simulink model "os_polyphase_synth_4096c"  (R2021a)
settings: Solver=VariableStepAuto  StartTime=0.0  StopTime=2304  RelTol=1e-3
undefined symbols (not defined in the file or in its folder): A, B, C, pfb_coeff_gen_calc
libraries: xbsIndex_r4 x22061, casper_library_misc x266, casper_library_delays x127, casper_library_multipliers x88
(part 109 of 241)

== system os_polyphase_synth_4096c/pfb_fir/pol1_in1_tap88 ==
  din : Inport
  sync : Inport  Port=2
  coeff : Inport  Port=3
  taps : Inport  Port=4
  Convert : xbsIndex_r4/Convert  gui_display_data_type=Fixed-point  arith_type="Signed  (2's comp)"  n_bits=36+3  bin_pt=34  float_type=Single  exp_bits=8  fraction_bits=24  quantization=Truncate  overflow=Wrap  en=off  latency=0  pipeline=off  dbl_ovrd=off  block_type=convert
  Convert1 : xbsIndex_r4/Convert  gui_display_data_type=Fixed-point  arith_type="Signed  (2's comp)"  n_bits=36+3  bin_pt=34  float_type=Single  exp_bits=8  fraction_bits=24  quantization=Truncate  overflow=Wrap  en=off  latency=0  pipeline=off  dbl_ovrd=off  block_type=convert
  DSP Macro 1.0 : xbsIndex_r4/DSP Macro 1.0   dsptype=Target XtremeDSP Slice: DSP48E2  instruction_list=A*B+C  AvailableInstructions=|  show_filtered=off  pipeline_options=Expert  dspimage=?  tier_1=off  tier_2=off  tier_3=off  tier_4=off  tier_5=off  tier_6=off  dreg_1=off  dreg_2=off  dreg_3=off  areg_1=off  areg_2=off  areg_3=on  areg_4=on  breg_1=off  breg_2=off  breg_3=off  breg_4=on  mreg_5=on  concatreg_3=off  concatreg_4=off  concatreg_5=off  creg_1=off  creg_2=off  creg_3=off  creg_4=off  creg_5=on  preg_6=off  cinreg_1=off  cinreg_2=off  cinreg_3=off  cinreg_4=off  cinreg_5=off  opreg_1=off  opreg_2=off  opreg_3=off  opreg_4=off  opreg_5=off  output_properties=Full_Precision  p_width=37  p_binarywidth=34  has_acout=off  has_bcout=off  has_pcout=off  has_carryout=off  has_carrycascout=off  has_ce=off  has_d_ce=off  has_a_ce=off  has_b_ce=off  has_concat_ce=off  has_c_ce=off  has_m_ce=off  has_p_ce=off  has_sel_ce=off  has_sclr=off  has_d_sclr=off  has_a_sclr=off  has_b_sclr=off  has_concat_sclr=off  has_c_sclr=off  has_m_sclr=off  has_p_sclr=off  has_sel_sclr=off  use_dsp48=on  simulation_type=xsim  gui_behaviour=Sysgen  instruction1='#'  ip_name=DSP Macro  ip_version=1.0  dsptool_ready=true  ipcore_usecache=true  ipcore_useipmodelcache=true  wrapper_available=true  port_translation_map={ 'ce' => 'en', 'sclr' => 'rst'}  ipcore_latency_function='has_combinational'  structural_sim=false  ipcore_verbose=false  ipcore_port_config={ 'carryin' => { 'arith' => 'ufix', 'binpt' => 0.00000000000, 'width' => 1.00000000000}, 'carrycascin' => { 'arith' => 'ufix', 'binpt' => 0.00000000000, 'width' => 1.00000000000}, 'carrycascout' => { 'arith' => 'ufix', 'binpt' => 0.00000000000, 'width' => 1.00000000000}, 'carryout' => { 'arith' => 'ufix', 'binpt' => 0.00000000000, 'width' => 1.00000000000}}  ipcore_xco_need_fpga_part=true  block_type=dsp_macro_v1_0
      | #
      | (A+D)
      | (A+D)*(A+D)
      | (A+D)*(A+D)+C
      | (A+D)*(A+D)+C+C
      | (A+D)*(A+D)+C+C+CARRYIN
      | (A+D)*(A+D)+C+CARRYIN
      | (A+D)*(A+D)+C+P
      | (A+D)*(A+D)+C+P+CARRYIN
      | (A+D)*(A+D)+C+P>>17
      | (A+D)*(A+D)+C+P>>17+CARRYIN
      | (A+D)*(A+D)+C+PCIN
      | (A+D)*(A+D)+C+PCIN+CARRYIN
      | (A+D)*(A+D)+C+PCIN>>17
      | (A+D)*(A+D)+C+PCIN>>17+CARRYIN
      | (A+D)*(A+D)+C-C
      | (A+D)*(A+D)+C-P
      | (A+D)*(A+D)+C-P>>17
      | (A+D)*(A+D)+C-PCIN
      | (A+D)*(A+D)+C-PCIN>>17
      | (A+D)*(A+D)+CARRYIN
      | (A+D)*(A+D)+P
      | (A+D)*(A+D)+P+C
      | (A+D)*(A+D)+P+C+CARRYIN
      | (A+D)*(A+D)+P+CARRYIN
      | (A+D)*(A+D)+P+P
      | (A+D)*(A+D)+P+P+CARRYIN
      | (A+D)*(A+D)+P+P>>17
      | (A+D)*(A+D)+P+P>>17+CARRYIN
      | (A+D)*(A+D)+P+PCIN
      | (A+D)*(A+D)+P+PCIN+CARRYIN
      | (A+D)*(A+D)+P+PCIN>>17
      | (A+D)*(A+D)+P+PCIN>>17+CARRYIN
      | (A+D)*(A+D)+P-C
      | (A+D)*(A+D)+P-P
      | (A+D)*(A+D)+P-P>>17
      | (A+D)*(A+D)+P-PCIN
      | (A+D)*(A+D)+P-PCIN>>17
      | (A+D)*(A+D)+P>>17
      | (A+D)*(A+D)+P>>17+C
      | (A+D)*(A+D)+P>>17+C+CARRYIN
      | (A+D)*(A+D)+P>>17+CARRYIN
      | (A+D)*(A+D)+P>>17+P
      | (A+D)*(A+D)+P>>17+P+CARRYIN
      | (A+D)*(A+D)+PCIN
      | (A+D)*(A+D)+PCIN+C
      | (A+D)*(A+D)+PCIN+C+CARRYIN
      | (A+D)*(A+D)+PCIN+CARRYIN
      | (A+D)*(A+D)+PCIN+P
      | (A+D)*(A+D)+PCIN+P+CARRYIN
      | (A+D)*(A+D)+PCIN>>17
      | (A+D)*(A+D)+PCIN>>17+C
      | (A+D)*(A+D)+PCIN>>17+C+CARRYIN
      | (A+D)*(A+D)+PCIN>>17+CARRYIN
      | (A+D)*(A+D)+PCIN>>17+P
      | (A+D)*(A+D)+PCIN>>17+P+CARRYIN
      | (A+D)*(A+D)-C
      | (A+D)*(A+D)-P
      | (A+D)*(A+D)-P>>17
      | (A+D)*(A+D)-PCIN
      | (A+D)*(A+D)-PCIN>>17
      | (A+D)*B
      | (A+D)*B+C
      | (A+D)*B+C+C
      | (A+D)*B+C+C+CARRYIN
      | (A+D)*B+C+CARRYIN
      | (A+D)*B+C+P
      | (A+D)*B+C+P+CARRYIN
      | (A+D)*B+C+P>>17
      | (A+D)*B+C+P>>17+CARRYIN
      | (A+D)*B+C+PCIN
      | (A+D)*B+C+PCIN+CARRYIN
      | (A+D)*B+C+PCIN>>17
      | (A+D)*B+C+PCIN>>17+CARRYIN
      | (A+D)*B+C-C
      | (A+D)*B+C-P
      | (A+D)*B+C-P>>17
      | (A+D)*B+C-PCIN
      | (A+D)*B+C-PCIN>>17
      | (A+D)*B+CARRYIN
      | (A+D)*B+P
      | (A+D)*B+P+C
      | (A+D)*B+P+C+CARRYIN
      | (A+D)*B+P+CARRYIN
      | (A+D)*B+P+P
      | (A+D)*B+P+P+CARRYIN
      | (A+D)*B+P+P>>17
      | (A+D)*B+P+P>>17+CARRYIN
      | (A+D)*B+P+PCIN
      | (A+D)*B+P+PCIN+CARRYIN
      | (A+D)*B+P+PCIN>>17
      | (A+D)*B+P+PCIN>>17+CARRYIN
      | (A+D)*B+P-C
      | (A+D)*B+P-P
      | (A+D)*B+P-P>>17
      | (A+D)*B+P-PCIN
      | (A+D)*B+P-PCIN>>17
      | (A+D)*B+P>>17
      | (A+D)*B+P>>17+C
      | (A+D)*B+P>>17+C+CARRYIN
      | (A+D)*B+P>>17+CARRYIN
      | (A+D)*B+P>>17+P
      | (A+D)*B+P>>17+P+CARRYIN
      | (A+D)*B+PCIN
      | (A+D)*B+PCIN+C
      | (A+D)*B+PCIN+C+CARRYIN
      | (A+D)*B+PCIN+CARRYIN
      | (A+D)*B+PCIN+P
      | (A+D)*B+PCIN+P+CARRYIN
      | (A+D)*B+PCIN>>17
      | (A+D)*B+PCIN>>17+C
      | (A+D)*B+PCIN>>17+C+CARRYIN
      | (A+D)*B+PCIN>>17+CARRYIN
      | (A+D)*B+PCIN>>17+P
      | (A+D)*B+PCIN>>17+P+CARRYIN
      | (A+D)*B-C
      | (A+D)*B-P
      | (A+D)*B-P>>17
      | (A+D)*B-PCIN
      | (A+D)*B-PCIN>>17
      | (A+D)*BCIN
      | (A+D)*BCIN+C
      | (A+D)*BCIN+C+C
      | (A+D)*BCIN+C+C+CARRYIN
      | (A+D)*BCIN+C+CARRYIN
      | (A+D)*BCIN+C+P
      | (A+D)*BCIN+C+P+CARRYIN
      | (A+D)*BCIN+C+P>>17
      | (A+D)*BCIN+C+P>>17+CARRYIN
      | (A+D)*BCIN+C+PCIN
      | (A+D)*BCIN+C+PCIN+CARRYIN
      | (A+D)*BCIN+C+PCIN>>17
      | (A+D)*BCIN+C+PCIN>>17+CARRYIN
      | (A+D)*BCIN+C-C
      | (A+D)*BCIN+C-P
      | (A+D)*BCIN+C-P>>17
      | (A+D)*BCIN+C-PCIN
      | (A+D)*BCIN+C-PCIN>>17
      | (A+D)*BCIN+CARRYIN
      | (A+D)*BCIN+P
      | (A+D)*BCIN+P+C
      | (A+D)*BCIN+P+C+CARRYIN
      | (A+D)*BCIN+P+CARRYIN
      | (A+D)*BCIN+P+P
      | (A+D)*BCIN+P+P+CARRYIN
      | (A+D)*BCIN+P+P>>17
      | (A+D)*BCIN+P+P>>17+CARRYIN
      | (A+D)*BCIN+P+PCIN
      | (A+D)*BCIN+P+PCIN+CARRYIN
      | (A+D)*BCIN+P+PCIN>>17
      | (A+D)*BCIN+P+PCIN>>17+CARRYIN
      | (A+D)*BCIN+P-C
      | (A+D)*BCIN+P-P
      | (A+D)*BCIN+P-P>>17
      | (A+D)*BCIN+P-PCIN
      | (A+D)*BCIN+P-PCIN>>17
      | (A+D)*BCIN+P>>17
      | (A+D)*BCIN+P>>17+C
      | (A+D)*BCIN+P>>17+C+CARRYIN
      | (A+D)*BCIN+P>>17+CARRYIN
      | (A+D)*BCIN+P>>17+P
      | (A+D)*BCIN+P>>17+P+CARRYIN
      | (A+D)*BCIN+PCIN
      | (A+D)*BCIN+PCIN+C
      | (A+D)*BCIN+PCIN+C+CARRYIN
      | (A+D)*BCIN+PCIN+CARRYIN
      | (A+D)*BCIN+PCIN+P
      | (A+D)*BCIN+PCIN+P+CARRYIN
      | (A+D)*BCIN+PCIN>>17
      | (A+D)*BCIN+PCIN>>17+C
      | (A+D)*BCIN+PCIN>>17+C+CARRYIN
      | (A+D)*BCIN+PCIN>>17+CARRYIN
      | (A+D)*BCIN+PCIN>>17+P
      | (A+D)*BCIN+PCIN>>17+P+CARRYIN
      | (A+D)*BCIN-C
      | (A+D)*BCIN-P
      | (A+D)*BCIN-P>>17
      | (A+D)*BCIN-PCIN
      | (A+D)*BCIN-PCIN>>17
      | (A+D)+C
      | (A+D)+C+C
      | (A+D)+C+C+CARRYIN
      | (A+D)+C+CARRYIN
      | (A+D)+C+P
      | (A+D)+C+P+CARRYIN
      | (A+D)+C+P>>17
      | (A+D)+C+P>>17+CARRYIN
      | (A+D)+C+PCIN
      | (A+D)+C+PCIN+CARRYIN
      | (A+D)+C+PCIN>>17
      | (A+D)+C+PCIN>>17+CARRYIN
      | (A+D)+C-C
      | (A+D)+C-P
      | (A+D)+C-P>>17
      | (A+D)+C-PCIN
      | (A+D)+C-PCIN>>17
      | (A+D)+CARRYIN
      | (A+D)+P
      | (A+D)+P+C
      | (A+D)+P+C+CARRYIN
      | (A+D)+P+CARRYIN
      | (A+D)+P+P
      | (A+D)+P+P+CARRYIN
      | (A+D)+P+P>>17
      | (A+D)+P+P>>17+CARRYIN
      | (A+D)+P+PCIN
      | (A+D)+P+PCIN+CARRYIN
      | (A+D)+P+PCIN>>17
      | (A+D)+P+PCIN>>17+CARRYIN
      | (A+D)+P-C
      | (A+D)+P-P
      | (A+D)+P-P>>17
      | (A+D)+P-PCIN
      | (A+D)+P-PCIN>>17
      | (A+D)+P>>17
      | (A+D)+P>>17+C
      | (A+D)+P>>17+C+CARRYIN
      | (A+D)+P>>17+CARRYIN
      | (A+D)+P>>17+P
      | (A+D)+P>>17+P+CARRYIN
      | (A+D)+PCIN
      | (A+D)+PCIN+C
      | (A+D)+PCIN+C+CARRYIN
      | (A+D)+PCIN+CARRYIN
      | (A+D)+PCIN+P
      | (A+D)+PCIN+P+CARRYIN
      | (A+D)+PCIN>>17
      | (A+D)+PCIN>>17+C
      | (A+D)+PCIN>>17+C+CARRYIN
      | (A+D)+PCIN>>17+CARRYIN
      | (A+D)+PCIN>>17+P
      | (A+D)+PCIN>>17+P+CARRYIN
      | (A+D)-C
      | (A+D)-P
      | (A+D)-P>>17
      | (A+D)-PCIN
      | (A+D)-PCIN>>17
      | (ACIN+D)
      | (ACIN+D)*(ACIN+D)
      | (ACIN+D)*(ACIN+D)+C
      | (ACIN+D)*(ACIN+D)+C+C
      | (ACIN+D)*(ACIN+D)+C+C+CARRYIN
      | (ACIN+D)*(ACIN+D)+C+CARRYIN
      | (ACIN+D)*(ACIN+D)+C+P
      | (ACIN+D)*(ACIN+D)+C+P+CARRYIN
      | (ACIN+D)*(ACIN+D)+C+P>>17
      | (ACIN+D)*(ACIN+D)+C+P>>17+CARRYIN
      | (ACIN+D)*(ACIN+D)+C+PCIN
      | (ACIN+D)*(ACIN+D)+C+PCIN+CARRYIN
      | (ACIN+D)*(ACIN+D)+C+PCIN>>17
      | (ACIN+D)*(ACIN+D)+C+PCIN>>17+CARRYIN
      | (ACIN+D)*(ACIN+D)+C-C
      | (ACIN+D)*(ACIN+D)+C-P
      | (ACIN+D)*(ACIN+D)+C-P>>17
      | (ACIN+D)*(ACIN+D)+C-PCIN
      | (ACIN+D)*(ACIN+D)+C-PCIN>>17
      | (ACIN+D)*(ACIN+D)+CARRYIN
      | (ACIN+D)*(ACIN+D)+P
      | (ACIN+D)*(ACIN+D)+P+C
      | (ACIN+D)*(ACIN+D)+P+C+CARRYIN
      | (ACIN+D)*(ACIN+D)+P+CARRYIN
      | (ACIN+D)*(ACIN+D)+P+P
      | (ACIN+D)*(ACIN+D)+P+P+CARRYIN
      | (ACIN+D)*(ACIN+D)+P+P>>17
      | (ACIN+D)*(ACIN+D)+P+P>>17+CARRYIN
      | (ACIN+D)*(ACIN+D)+P+PCIN
      | (ACIN+D)*(ACIN+D)+P+PCIN+CARRYIN
      | (ACIN+D)*(ACIN+D)+P+PCIN>>17
      | (ACIN+D)*(ACIN+D)+P+PCIN>>17+CARRYIN
      | (ACIN+D)*(ACIN+D)+P-C
      | (ACIN+D)*(ACIN+D)+P-P
      | (ACIN+D)*(ACIN+D)+P-P>>17
      | (ACIN+D)*(ACIN+D)+P-PCIN
      | (ACIN+D)*(ACIN+D)+P-PCIN>>17
      | (ACIN+D)*(ACIN+D)+P>>17
      | (ACIN+D)*(ACIN+D)+P>>17+C
      | (ACIN+D)*(ACIN+D)+P>>17+C+CARRYIN
      | (ACIN+D)*(ACIN+D)+P>>17+CARRYIN
      | (ACIN+D)*(ACIN+D)+P>>17+P
      | (ACIN+D)*(ACIN+D)+P>>17+P+CARRYIN
      | (ACIN+D)*(ACIN+D)+PCIN
      | (ACIN+D)*(ACIN+D)+PCIN+C
      | (ACIN+D)*(ACIN+D)+PCIN+C+CARRYIN
      | (ACIN+D)*(ACIN+D)+PCIN+CARRYIN
      | (ACIN+D)*(ACIN+D)+PCIN+P
      | (ACIN+D)*(ACIN+D)+PCIN+P+CARRYIN
      | (ACIN+D)*(ACIN+D)+PCIN>>17
      | (ACIN+D)*(ACIN+D)+PCIN>>17+C
      | (ACIN+D)*(ACIN+D)+PCIN>>17+C+CARRYIN
      | (ACIN+D)*(ACIN+D)+PCIN>>17+CARRYIN
      | (ACIN+D)*(ACIN+D)+PCIN>>17+P
      | (ACIN+D)*(ACIN+D)+PCIN>>17+P+CARRYIN
      | (ACIN+D)*(ACIN+D)-C
      | (ACIN+D)*(ACIN+D)-P
      | (ACIN+D)*(ACIN+D)-P>>17
      | (ACIN+D)*(ACIN+D)-PCIN
      | (ACIN+D)*(ACIN+D)-PCIN>>17
      | (ACIN+D)*B
      | (ACIN+D)*B+C
      | (ACIN+D)*B+C+C
      | (ACIN+D)*B+C+C+CARRYIN
      | (ACIN+D)*B+C+CARRYIN
      | (ACIN+D)*B+C+P
      | (ACIN+D)*B+C+P+CARRYIN
      | (ACIN+D)*B+C+P>>17
      | (ACIN+D)*B+C+P>>17+CARRYIN
      | (ACIN+D)*B+C+PCIN
      | (ACIN+D)*B+C+PCIN+CARRYIN
      | (ACIN+D)*B+C+PCIN>>17
      | (ACIN+D)*B+C+PCIN>>17+CARRYIN
      | (ACIN+D)*B+C-C
      | (ACIN+D)*B+C-P
      | (ACIN+D)*B+C-P>>17
      | (ACIN+D)*B+C-PCIN
      | (ACIN+D)*B+C-PCIN>>17
      | (ACIN+D)*B+CARRYIN
      | (ACIN+D)*B+P
      | (ACIN+D)*B+P+C
      | (ACIN+D)*B+P+C+CARRYIN
      | (ACIN+D)*B+P+CARRYIN
      | (ACIN+D)*B+P+P
      | (ACIN+D)*B+P+P+CARRYIN
      | (ACIN+D)*B+P+P>>17
      | (ACIN+D)*B+P+P>>17+CARRYIN
      | (ACIN+D)*B+P+PCIN
      | (ACIN+D)*B+P+PCIN+CARRYIN
      | (ACIN+D)*B+P+PCIN>>17
      | (ACIN+D)*B+P+PCIN>>17+CARRYIN
      | (ACIN+D)*B+P-C
      | (ACIN+D)*B+P-P
      | (ACIN+D)*B+P-P>>17
      | (ACIN+D)*B+P-PCIN
      | (ACIN+D)*B+P-PCIN>>17
      | (ACIN+D)*B+P>>17
      | (ACIN+D)*B+P>>17+C
      | (ACIN+D)*B+P>>17+C+CARRYIN
      | (ACIN+D)*B+P>>17+CARRYIN
      | (ACIN+D)*B+P>>17+P
      | (ACIN+D)*B+P>>17+P+CARRYIN
      | (ACIN+D)*B+PCIN
      | (ACIN+D)*B+PCIN+C
      | (ACIN+D)*B+PCIN+C+CARRYIN
      | (ACIN+D)*B+PCIN+CARRYIN
      | (ACIN+D)*B+PCIN+P
      | (ACIN+D)*B+PCIN+P+CARRYIN
      | (ACIN+D)*B+PCIN>>17
      | (ACIN+D)*B+PCIN>>17+C
      | (ACIN+D)*B+PCIN>>17+C+CARRYIN
      | (ACIN+D)*B+PCIN>>17+CARRYIN
      | (ACIN+D)*B+PCIN>>17+P
      | (ACIN+D)*B+PCIN>>17+P+CARRYIN
      | (ACIN+D)*B-C
      | (ACIN+D)*B-P
      | (ACIN+D)*B-P>>17
      | (ACIN+D)*B-PCIN
      | (ACIN+D)*B-PCIN>>17
      | (ACIN+D)*BCIN
      | (ACIN+D)*BCIN+C
      | (ACIN+D)*BCIN+C+C
      | (ACIN+D)*BCIN+C+C+CARRYIN
      | (ACIN+D)*BCIN+C+CARRYIN
      | (ACIN+D)*BCIN+C+P
      | (ACIN+D)*BCIN+C+P+CARRYIN
      | (ACIN+D)*BCIN+C+P>>17
      | (ACIN+D)*BCIN+C+P>>17+CARRYIN
      | (ACIN+D)*BCIN+C+PCIN
      | (ACIN+D)*BCIN+C+PCIN+CARRYIN
      | (ACIN+D)*BCIN+C+PCIN>>17
      | (ACIN+D)*BCIN+C+PCIN>>17+CARRYIN
      | (ACIN+D)*BCIN+C-C
      | (ACIN+D)*BCIN+C-P
      | (ACIN+D)*BCIN+C-P>>17
      | (ACIN+D)*BCIN+C-PCIN
      | (ACIN+D)*BCIN+C-PCIN>>17
      | (ACIN+D)*BCIN+CARRYIN
      | (ACIN+D)*BCIN+P
      | (ACIN+D)*BCIN+P+C
      | (ACIN+D)*BCIN+P+C+CARRYIN
      | (ACIN+D)*BCIN+P+CARRYIN
      | (ACIN+D)*BCIN+P+P
      | (ACIN+D)*BCIN+P+P+CARRYIN
      | (ACIN+D)*BCIN+P+P>>17
      | (ACIN+D)*BCIN+P+P>>17+CARRYIN
      | (ACIN+D)*BCIN+P+PCIN
      | (ACIN+D)*BCIN+P+PCIN+CARRYIN
      | (ACIN+D)*BCIN+P+PCIN>>17
      | (ACIN+D)*BCIN+P+PCIN>>17+CARRYIN
      | (ACIN+D)*BCIN+P-C
      | (ACIN+D)*BCIN+P-P
      | (ACIN+D)*BCIN+P-P>>17
      | (ACIN+D)*BCIN+P-PCIN
      | (ACIN+D)*BCIN+P-PCIN>>17
      | (ACIN+D)*BCIN+P>>17
      | (ACIN+D)*BCIN+P>>17+C
      | (ACIN+D)*BCIN+P>>17+C+CARRYIN
      | (ACIN+D)*BCIN+P>>17+CARRYIN
      | (ACIN+D)*BCIN+P>>17+P
      | (ACIN+D)*BCIN+P>>17+P+CARRYIN
      | (ACIN+D)*BCIN+PCIN
      | (ACIN+D)*BCIN+PCIN+C
      | (ACIN+D)*BCIN+PCIN+C+CARRYIN
      | (ACIN+D)*BCIN+PCIN+CARRYIN
      | (ACIN+D)*BCIN+PCIN+P
      | (ACIN+D)*BCIN+PCIN+P+CARRYIN
      | (ACIN+D)*BCIN+PCIN>>17
      | (ACIN+D)*BCIN+PCIN>>17+C
      | (ACIN+D)*BCIN+PCIN>>17+C+CARRYIN
      | (ACIN+D)*BCIN+PCIN>>17+CARRYIN
      | (ACIN+D)*BCIN+PCIN>>17+P
      | (ACIN+D)*BCIN+PCIN>>17+P+CARRYIN
      | (ACIN+D)*BCIN-C
      | (ACIN+D)*BCIN-P
      | (ACIN+D)*BCIN-P>>17
      | (ACIN+D)*BCIN-PCIN
      | (ACIN+D)*BCIN-PCIN>>17
      | (ACIN+D)+C
      | (ACIN+D)+C+C
      | (ACIN+D)+C+C+CARRYIN
      | (ACIN+D)+C+CARRYIN
      | (ACIN+D)+C+P
      | (ACIN+D)+C+P+CARRYIN
      | (ACIN+D)+C+P>>17
      | (ACIN+D)+C+P>>17+CARRYIN
      | (ACIN+D)+C+PCIN
      | (ACIN+D)+C+PCIN+CARRYIN
      | (ACIN+D)+C+PCIN>>17
      | (ACIN+D)+C+PCIN>>17+CARRYIN
      | (ACIN+D)+C-C
      | (ACIN+D)+C-P
      | (ACIN+D)+C-P>>17
      | (ACIN+D)+C-PCIN
      | (ACIN+D)+C-PCIN>>17
      | (ACIN+D)+CARRYIN
      | (ACIN+D)+P
      | (ACIN+D)+P+C
      | (ACIN+D)+P+C+CARRYIN
      | (ACIN+D)+P+CARRYIN
      | (ACIN+D)+P+P
      | (ACIN+D)+P+P+CARRYIN
      | (ACIN+D)+P+P>>17
      | (ACIN+D)+P+P>>17+CARRYIN
      | (ACIN+D)+P+PCIN
      | (ACIN+D)+P+PCIN+CARRYIN
      | (ACIN+D)+P+PCIN>>17
      | (ACIN+D)+P+PCIN>>17+CARRYIN
      | (ACIN+D)+P-C
      | (ACIN+D)+P-P
      | (ACIN+D)+P-P>>17
      | (ACIN+D)+P-PCIN
      | (ACIN+D)+P-PCIN>>17
      | (ACIN+D)+P>>17
      | (ACIN+D)+P>>17+C
      | (ACIN+D)+P>>17+C+CARRYIN
      | (ACIN+D)+P>>17+CARRYIN
      | (ACIN+D)+P>>17+P
      | (ACIN+D)+P>>17+P+CARRYIN
      | (ACIN+D)+PCIN
      | (ACIN+D)+PCIN+C
      | (ACIN+D)+PCIN+C+CARRYIN
      | (ACIN+D)+PCIN+CARRYIN
      | (ACIN+D)+PCIN+P
      | (ACIN+D)+PCIN+P+CARRYIN
      | (ACIN+D)+PCIN>>17
      | (ACIN+D)+PCIN>>17+C
      | (ACIN+D)+PCIN>>17+C+CARRYIN
      | (ACIN+D)+PCIN>>17+CARRYIN
      | (ACIN+D)+PCIN>>17+P
      | (ACIN+D)+PCIN>>17+P+CARRYIN
      | (ACIN+D)-C
      | (ACIN+D)-P
      | (ACIN+D)-P>>17
      | (ACIN+D)-PCIN
      | (ACIN+D)-PCIN>>17
      | (D+A)
      | (D+A)*(D+A)
      | (D+A)*(D+A)+C
      | (D+A)*(D+A)+C+C
      | (D+A)*(D+A)+C+C+CARRYIN
      | (D+A)*(D+A)+C+CARRYIN
      | (D+A)*(D+A)+C+P
      | (D+A)*(D+A)+C+P+CARRYIN
      | (D+A)*(D+A)+C+P>>17
      | (D+A)*(D+A)+C+P>>17+CARRYIN
      | (D+A)*(D+A)+C+PCIN
      | (D+A)*(D+A)+C+PCIN+CARRYIN
      | (D+A)*(D+A)+C+PCIN>>17
      | (D+A)*(D+A)+C+PCIN>>17+CARRYIN
      | (D+A)*(D+A)+C-C
      | (D+A)*(D+A)+C-P
      | (D+A)*(D+A)+C-P>>17
      | (D+A)*(D+A)+C-PCIN
      | (D+A)*(D+A)+C-PCIN>>17
      | (D+A)*(D+A)+CARRYIN
      | (D+A)*(D+A)+P
      | (D+A)*(D+A)+P+C
      | (D+A)*(D+A)+P+C+CARRYIN
      | (D+A)*(D+A)+P+CARRYIN
      | (D+A)*(D+A)+P+P
      | (D+A)*(D+A)+P+P+CARRYIN
      | (D+A)*(D+A)+P+P>>17
      | (D+A)*(D+A)+P+P>>17+CARRYIN
      | (D+A)*(D+A)+P+PCIN
      | (D+A)*(D+A)+P+PCIN+CARRYIN
      | (D+A)*(D+A)+P+PCIN>>17
      | (D+A)*(D+A)+P+PCIN>>17+CARRYIN
      | (D+A)*(D+A)+P-C
      | (D+A)*(D+A)+P-P
      | (D+A)*(D+A)+P-P>>17
      | (D+A)*(D+A)+P-PCIN
      | (D+A)*(D+A)+P-PCIN>>17
      | (D+A)*(D+A)+P>>17
      | (D+A)*(D+A)+P>>17+C
      | (D+A)*(D+A)+P>>17+C+CARRYIN
      | (D+A)*(D+A)+P>>17+CARRYIN
      | (D+A)*(D+A)+P>>17+P
      | (D+A)*(D+A)+P>>17+P+CARRYIN
      | (D+A)*(D+A)+PCIN
      | (D+A)*(D+A)+PCIN+C
      | (D+A)*(D+A)+PCIN+C+CARRYIN
      | (D+A)*(D+A)+PCIN+CARRYIN
      | (D+A)*(D+A)+PCIN+P
      | (D+A)*(D+A)+PCIN+P+CARRYIN
      | (D+A)*(D+A)+PCIN>>17
      | (D+A)*(D+A)+PCIN>>17+C
      | (D+A)*(D+A)+PCIN>>17+C+CARRYIN
      | (D+A)*(D+A)+PCIN>>17+CARRYIN
      | (D+A)*(D+A)+PCIN>>17+P
      | (D+A)*(D+A)+PCIN>>17+P+CARRYIN
      | (D+A)*(D+A)-C
      | (D+A)*(D+A)-P
      | (D+A)*(D+A)-P>>17
      | (D+A)*(D+A)-PCIN
      | (D+A)*(D+A)-PCIN>>17
      | (D+A)*B
      | (D+A)*B+C
      | (D+A)*B+C+C
      | (D+A)*B+C+C+CARRYIN
      | (D+A)*B+C+CARRYIN
      | (D+A)*B+C+P
      | (D+A)*B+C+P+CARRYIN
      | (D+A)*B+C+P>>17
      | (D+A)*B+C+P>>17+CARRYIN
      | (D+A)*B+C+PCIN
      | (D+A)*B+C+PCIN+CARRYIN
      | (D+A)*B+C+PCIN>>17
      | (D+A)*B+C+PCIN>>17+CARRYIN
      | (D+A)*B+C-C
      | (D+A)*B+C-P
      | (D+A)*B+C-P>>17
      | (D+A)*B+C-PCIN
      | (D+A)*B+C-PCIN>>17
      | (D+A)*B+CARRYIN
      | (D+A)*B+P
      | (D+A)*B+P+C
      | (D+A)*B+P+C+CARRYIN
      | (D+A)*B+P+CARRYIN
      | (D+A)*B+P+P
      | (D+A)*B+P+P+CARRYIN
      | (D+A)*B+P+P>>17
      | (D+A)*B+P+P>>17+CARRYIN
      | (D+A)*B+P+PCIN
      | (D+A)*B+P+PCIN+CARRYIN
      | (D+A)*B+P+PCIN>>17
      | (D+A)*B+P+PCIN>>17+CARRYIN
      | (D+A)*B+P-C
      | (D+A)*B+P-P
      | (D+A)*B+P-P>>17
      | (D+A)*B+P-PCIN
      | (D+A)*B+P-PCIN>>17
      | (D+A)*B+P>>17
      | (D+A)*B+P>>17+C
      | (D+A)*B+P>>17+C+CARRYIN
      | (D+A)*B+P>>17+CARRYIN
      | (D+A)*B+P>>17+P
      | (D+A)*B+P>>17+P+CARRYIN
      | (D+A)*B+PCIN
      | (D+A)*B+PCIN+C
      | (D+A)*B+PCIN+C+CARRYIN
      | (D+A)*B+PCIN+CARRYIN
      | (D+A)*B+PCIN+P
      | (D+A)*B+PCIN+P+CARRYIN
      | (D+A)*B+PCIN>>17
      | (D+A)*B+PCIN>>17+C
      | (D+A)*B+PCIN>>17+C+CARRYIN
      | (D+A)*B+PCIN>>17+CARRYIN
      | (D+A)*B+PCIN>>17+P
      | (D+A)*B+PCIN>>17+P+CARRYIN
      | (D+A)*B-C
      | (D+A)*B-P
      | (D+A)*B-P>>17
      | (D+A)*B-PCIN
      | (D+A)*B-PCIN>>17
      | (D+A)*BCIN
      | (D+A)*BCIN+C
      | (D+A)*BCIN+C+C
      | (D+A)*BCIN+C+C+CARRYIN
      | (D+A)*BCIN+C+CARRYIN
      | (D+A)*BCIN+C+P
      | (D+A)*BCIN+C+P+CARRYIN
      | (D+A)*BCIN+C+P>>17
      | (D+A)*BCIN+C+P>>17+CARRYIN
      | (D+A)*BCIN+C+PCIN
      | (D+A)*BCIN+C+PCIN+CARRYIN
      | (D+A)*BCIN+C+PCIN>>17
      | (D+A)*BCIN+C+PCIN>>17+CARRYIN
      | (D+A)*BCIN+C-C
      | (D+A)*BCIN+C-P
      | (D+A)*BCIN+C-P>>17
      | (D+A)*BCIN+C-PCIN
      | (D+A)*BCIN+C-PCIN>>17
      | (D+A)*BCIN+CARRYIN
      | (D+A)*BCIN+P
      | (D+A)*BCIN+P+C
      | (D+A)*BCIN+P+C+CARRYIN
      | (D+A)*BCIN+P+CARRYIN
      | (D+A)*BCIN+P+P
      | (D+A)*BCIN+P+P+CARRYIN
      | (D+A)*BCIN+P+P>>17
      | (D+A)*BCIN+P+P>>17+CARRYIN
      | (D+A)*BCIN+P+PCIN
      | (D+A)*BCIN+P+PCIN+CARRYIN
      | (D+A)*BCIN+P+PCIN>>17
      | (D+A)*BCIN+P+PCIN>>17+CARRYIN
      | (D+A)*BCIN+P-C
      | (D+A)*BCIN+P-P
      | (D+A)*BCIN+P-P>>17
      | (D+A)*BCIN+P-PCIN
      | (D+A)*BCIN+P-PCIN>>17
      | (D+A)*BCIN+P>>17
      | (D+A)*BCIN+P>>17+C
      | (D+A)*BCIN+P>>17+C+CARRYIN
      | (D+A)*BCIN+P>>17+CARRYIN
      | (D+A)*BCIN+P>>17+P
      | (D+A)*BCIN+P>>17+P+CARRYIN
      | (D+A)*BCIN+PCIN
      | (D+A)*BCIN+PCIN+C
      | (D+A)*BCIN+PCIN+C+CARRYIN
      | (D+A)*BCIN+PCIN+CARRYIN
      | (D+A)*BCIN+PCIN+P
      | (D+A)*BCIN+PCIN+P+CARRYIN
      | (D+A)*BCIN+PCIN>>17
      | (D+A)*BCIN+PCIN>>17+C
      | (D+A)*BCIN+PCIN>>17+C+CARRYIN
      | (D+A)*BCIN+PCIN>>17+CARRYIN
      | (D+A)*BCIN+PCIN>>17+P
      | (D+A)*BCIN+PCIN>>17+P+CARRYIN
      | (D+A)*BCIN-C
      | (D+A)*BCIN-P
      | (D+A)*BCIN-P>>17
      | (D+A)*BCIN-PCIN
      | (D+A)*BCIN-PCIN>>17
      | (D+A)+C
      | (D+A)+C+C
      | (D+A)+C+C+CARRYIN
      | (D+A)+C+CARRYIN
      | (D+A)+C+P
      | (D+A)+C+P+CARRYIN
      | (D+A)+C+P>>17
      | (D+A)+C+P>>17+CARRYIN
      | (D+A)+C+PCIN
      | (D+A)+C+PCIN+CARRYIN
      | (D+A)+C+PCIN>>17
      | (D+A)+C+PCIN>>17+CARRYIN
      | (D+A)+C-C
      | (D+A)+C-P
      | (D+A)+C-P>>17
      | (D+A)+C-PCIN
      | (D+A)+C-PCIN>>17
      | (D+A)+CARRYIN
      | (D+A)+P
      | (D+A)+P+C
      | (D+A)+P+C+CARRYIN
      | (D+A)+P+CARRYIN
      | (D+A)+P+P
      | (D+A)+P+P+CARRYIN
      | (D+A)+P+P>>17
      | (D+A)+P+P>>17+CARRYIN
      | (D+A)+P+PCIN
      | (D+A)+P+PCIN+CARRYIN
      | (D+A)+P+PCIN>>17
      | (D+A)+P+PCIN>>17+CARRYIN
      | (D+A)+P-C
      | (D+A)+P-P
      | (D+A)+P-P>>17
      | (D+A)+P-PCIN
      | (D+A)+P-PCIN>>17
      | (D+A)+P>>17
      | (D+A)+P>>17+C
      | (D+A)+P>>17+C+CARRYIN
      | (D+A)+P>>17+CARRYIN
      | (D+A)+P>>17+P
      | (D+A)+P>>17+P+CARRYIN
      | (D+A)+PCIN
      | (D+A)+PCIN+C
      | (D+A)+PCIN+C+CARRYIN
      | (D+A)+PCIN+CARRYIN
      | (D+A)+PCIN+P
      | (D+A)+PCIN+P+CARRYIN
      | (D+A)+PCIN>>17
      | (D+A)+PCIN>>17+C
      | (D+A)+PCIN>>17+C+CARRYIN
      | (D+A)+PCIN>>17+CARRYIN
      | (D+A)+PCIN>>17+P
      | (D+A)+PCIN>>17+P+CARRYIN
      | (D+A)-C
      | (D+A)-P
      | (D+A)-P>>17
      | (D+A)-PCIN
      | (D+A)-PCIN>>17
      | (D+ACIN)
      | (D+ACIN)*(D+ACIN)
      | (D+ACIN)*(D+ACIN)+C
      | (D+ACIN)*(D+ACIN)+C+C
      | (D+ACIN)*(D+ACIN)+C+C+CARRYIN
      | (D+ACIN)*(D+ACIN)+C+CARRYIN
      | (D+ACIN)*(D+ACIN)+C+P
      | (D+ACIN)*(D+ACIN)+C+P+CARRYIN
      | (D+ACIN)*(D+ACIN)+C+P>>17
      | (D+ACIN)*(D+ACIN)+C+P>>17+CARRYIN
      | (D+ACIN)*(D+ACIN)+C+PCIN
      | (D+ACIN)*(D+ACIN)+C+PCIN+CARRYIN
      | (D+ACIN)*(D+ACIN)+C+PCIN>>17
      | (D+ACIN)*(D+ACIN)+C+PCIN>>17+CARRYIN
      | (D+ACIN)*(D+ACIN)+C-C
      | (D+ACIN)*(D+ACIN)+C-P
      | (D+ACIN)*(D+ACIN)+C-P>>17
      | (D+ACIN)*(D+ACIN)+C-PCIN
      | (D+ACIN)*(D+ACIN)+C-PCIN>>17
      | (D+ACIN)*(D+ACIN)+CARRYIN
      | (D+ACIN)*(D+ACIN)+P
      | (D+ACIN)*(D+ACIN)+P+C
      | (D+ACIN)*(D+ACIN)+P+C+CARRYIN
      | (D+ACIN)*(D+ACIN)+P+CARRYIN
      | (D+ACIN)*(D+ACIN)+P+P
      | (D+ACIN)*(D+ACIN)+P+P+CARRYIN
      | (D+ACIN)*(D+ACIN)+P+P>>17
      | (D+ACIN)*(D+ACIN)+P+P>>17+CARRYIN
      | (D+ACIN)*(D+ACIN)+P+PCIN
      | (D+ACIN)*(D+ACIN)+P+PCIN+CARRYIN
      | (D+ACIN)*(D+ACIN)+P+PCIN>>17
      | (D+ACIN)*(D+ACIN)+P+PCIN>>17+CARRYIN
      | (D+ACIN)*(D+ACIN)+P-C
      | (D+ACIN)*(D+ACIN)+P-P
      | (D+ACIN)*(D+ACIN)+P-P>>17
      | (D+ACIN)*(D+ACIN)+P-PCIN
      | (D+ACIN)*(D+ACIN)+P-PCIN>>17
      | (D+ACIN)*(D+ACIN)+P>>17
      | (D+ACIN)*(D+ACIN)+P>>17+C
      | (D+ACIN)*(D+ACIN)+P>>17+C+CARRYIN
      | (D+ACIN)*(D+ACIN)+P>>17+CARRYIN
      | (D+ACIN)*(D+ACIN)+P>>17+P
      | (D+ACIN)*(D+ACIN)+P>>17+P+CARRYIN
      | (D+ACIN)*(D+ACIN)+PCIN
      | (D+ACIN)*(D+ACIN)+PCIN+C
      | (D+ACIN)*(D+ACIN)+PCIN+C+CARRYIN
      | (D+ACIN)*(D+ACIN)+PCIN+CARRYIN
      | (D+ACIN)*(D+ACIN)+PCIN+P
      | (D+ACIN)*(D+ACIN)+PCIN+P+CARRYIN
      | (D+ACIN)*(D+ACIN)+PCIN>>17
      | (D+ACIN)*(D+ACIN)+PCIN>>17+C
      | (D+ACIN)*(D+ACIN)+PCIN>>17+C+CARRYIN
      | (D+ACIN)*(D+ACIN)+PCIN>>17+CARRYIN
      | (D+ACIN)*(D+ACIN)+PCIN>>17+P
      | (D+ACIN)*(D+ACIN)+PCIN>>17+P+CARRYIN
      | (D+ACIN)*(D+ACIN)-C
      | (D+ACIN)*(D+ACIN)-P
      | (D+ACIN)*(D+ACIN)-P>>17
      | (D+ACIN)*(D+ACIN)-PCIN
      | (D+ACIN)*(D+ACIN)-PCIN>>17
      | (D+ACIN)*B
      | (D+ACIN)*B+C
      | (D+ACIN)*B+C+C
      | (D+ACIN)*B+C+C+CARRYIN
      | (D+ACIN)*B+C+CARRYIN
      | (D+ACIN)*B+C+P
      | (D+ACIN)*B+C+P+CARRYIN
      | (D+ACIN)*B+C+P>>17
      | (D+ACIN)*B+C+P>>17+CARRYIN
      | (D+ACIN)*B+C+PCIN
      | (D+ACIN)*B+C+PCIN+CARRYIN
      | (D+ACIN)*B+C+PCIN>>17
      | (D+ACIN)*B+C+PCIN>>17+CARRYIN
      | (D+ACIN)*B+C-C
      | (D+ACIN)*B+C-P
      | (D+ACIN)*B+C-P>>17
      | (D+ACIN)*B+C-PCIN
      | (D+ACIN)*B+C-PCIN>>17
      | (D+ACIN)*B+CARRYIN
      | (D+ACIN)*B+P
      | (D+ACIN)*B+P+C
      | (D+ACIN)*B+P+C+CARRYIN
      | (D+ACIN)*B+P+CARRYIN
      | (D+ACIN)*B+P+P
      | (D+ACIN)*B+P+P+CARRYIN
      | (D+ACIN)*B+P+P>>17
      | (D+ACIN)*B+P+P>>17+CARRYIN
      | (D+ACIN)*B+P+PCIN
      | (D+ACIN)*B+P+PCIN+CARRYIN
      | (D+ACIN)*B+P+PCIN>>17
      | (D+ACIN)*B+P+PCIN>>17+CARRYIN
      | (D+ACIN)*B+P-C
      | (D+ACIN)*B+P-P
      | (D+ACIN)*B+P-P>>17
      | (D+ACIN)*B+P-PCIN
      | (D+ACIN)*B+P-PCIN>>17
      | (D+ACIN)*B+P>>17
      | (D+ACIN)*B+P>>17+C
      | (D+ACIN)*B+P>>17+C+CARRYIN
      | (D+ACIN)*B+P>>17+CARRYIN
      | (D+ACIN)*B+P>>17+P
      | (D+ACIN)*B+P>>17+P+CARRYIN
      | (D+ACIN)*B+PCIN
      | (D+ACIN)*B+PCIN+C
      | (D+ACIN)*B+PCIN+C+CARRYIN
      | (D+ACIN)*B+PCIN+CARRYIN
      | (D+ACIN)*B+PCIN+P
      | (D+ACIN)*B+PCIN+P+CARRYIN
      | (D+ACIN)*B+PCIN>>17
      | (D+ACIN)*B+PCIN>>17+C
      | (D+ACIN)*B+PCIN>>17+C+CARRYIN
      | (D+ACIN)*B+PCIN>>17+CARRYIN
      | (D+ACIN)*B+PCIN>>17+P
      | (D+ACIN)*B+PCIN>>17+P+CARRYIN
      | (D+ACIN)*B-C
      | (D+ACIN)*B-P
      | (D+ACIN)*B-P>>17
      | (D+ACIN)*B-PCIN
      | (D+ACIN)*B-PCIN>>17
      | (D+ACIN)*BCIN
      | (D+ACIN)*BCIN+C
      | (D+ACIN)*BCIN+C+C
      | (D+ACIN)*BCIN+C+C+CARRYIN
      | (D+ACIN)*BCIN+C+CARRYIN
      | (D+ACIN)*BCIN+C+P
      | (D+ACIN)*BCIN+C+P+CARRYIN
      | (D+ACIN)*BCIN+C+P>>17
      | (D+ACIN)*BCIN+C+P>>17+CARRYIN
      | (D+ACIN)*BCIN+C+PCIN
      | (D+ACIN)*BCIN+C+PCIN+CARRYIN
      | (D+ACIN)*BCIN+C+PCIN>>17
      | (D+ACIN)*BCIN+C+PCIN>>17+CARRYIN
      | (D+ACIN)*BCIN+C-C
      | (D+ACIN)*BCIN+C-P
      | (D+ACIN)*BCIN+C-P>>17
      | (D+ACIN)*BCIN+C-PCIN
      | (D+ACIN)*BCIN+C-PCIN>>17
      | (D+ACIN)*BCIN+CARRYIN
      | (D+ACIN)*BCIN+P
      | (D+ACIN)*BCIN+P+C
      | (D+ACIN)*BCIN+P+C+CARRYIN
      | (D+ACIN)*BCIN+P+CARRYIN
      | (D+ACIN)*BCIN+P+P
      | (D+ACIN)*BCIN+P+P+CARRYIN
      | (D+ACIN)*BCIN+P+P>>17
      | (D+ACIN)*BCIN+P+P>>17+CARRYIN
      | (D+ACIN)*BCIN+P+PCIN
      | (D+ACIN)*BCIN+P+PCIN+CARRYIN
      | (D+ACIN)*BCIN+P+PCIN>>17
      | (D+ACIN)*BCIN+P+PCIN>>17+CARRYIN
      | (D+ACIN)*BCIN+P-C
      | (D+ACIN)*BCIN+P-P
      | (D+ACIN)*BCIN+P-P>>17
      | (D+ACIN)*BCIN+P-PCIN
      | (D+ACIN)*BCIN+P-PCIN>>17
      | (D+ACIN)*BCIN+P>>17
      | (D+ACIN)*BCIN+P>>17+C
      | (D+ACIN)*BCIN+P>>17+C+CARRYIN
      | (D+ACIN)*BCIN+P>>17+CARRYIN
      | (D+ACIN)*BCIN+P>>17+P
      | (D+ACIN)*BCIN+P>>17+P+CARRYIN
      | (D+ACIN)*BCIN+PCIN
      | (D+ACIN)*BCIN+PCIN+C
      | (D+ACIN)*BCIN+PCIN+C+CARRYIN
      | (D+ACIN)*BCIN+PCIN+CARRYIN
      | (D+ACIN)*BCIN+PCIN+P
      | (D+ACIN)*BCIN+PCIN+P+CARRYIN
      | (D+ACIN)*BCIN+PCIN>>17
      | (D+ACIN)*BCIN+PCIN>>17+C
      | (D+ACIN)*BCIN+PCIN>>17+C+CARRYIN
      | (D+ACIN)*BCIN+PCIN>>17+CARRYIN
      | (D+ACIN)*BCIN+PCIN>>17+P
      | (D+ACIN)*BCIN+PCIN>>17+P+CARRYIN
      | (D+ACIN)*BCIN-C
      | (D+ACIN)*BCIN-P
      | (D+ACIN)*BCIN-P>>17
      | (D+ACIN)*BCIN-PCIN
      | (D+ACIN)*BCIN-PCIN>>17
      | (D+ACIN)+C
      | (D+ACIN)+C+C
      | (D+ACIN)+C+C+CARRYIN
      | (D+ACIN)+C+CARRYIN
      | (D+ACIN)+C+P
      | (D+ACIN)+C+P+CARRYIN
      | (D+ACIN)+C+P>>17
      | (D+ACIN)+C+P>>17+CARRYIN
      | (D+ACIN)+C+PCIN
      | (D+ACIN)+C+PCIN+CARRYIN
      | (D+ACIN)+C+PCIN>>17
      | (D+ACIN)+C+PCIN>>17+CARRYIN
      | (D+ACIN)+C-C
      | (D+ACIN)+C-P
      | (D+ACIN)+C-P>>17
      | (D+ACIN)+C-PCIN
      | (D+ACIN)+C-PCIN>>17
      | (D+ACIN)+CARRYIN
      | (D+ACIN)+P
      | (D+ACIN)+P+C
      | (D+ACIN)+P+C+CARRYIN
      | (D+ACIN)+P+CARRYIN
      | (D+ACIN)+P+P
      | (D+ACIN)+P+P+CARRYIN
      | (D+ACIN)+P+P>>17
      | (D+ACIN)+P+P>>17+CARRYIN
      | (D+ACIN)+P+PCIN
      | (D+ACIN)+P+PCIN+CARRYIN
      | (D+ACIN)+P+PCIN>>17
      | (D+ACIN)+P+PCIN>>17+CARRYIN
      | (D+ACIN)+P-C
      | (D+ACIN)+P-P
      | (D+ACIN)+P-P>>17
      | (D+ACIN)+P-PCIN
      | (D+ACIN)+P-PCIN>>17
      | (D+ACIN)+P>>17
      | (D+ACIN)+P>>17+C
      | (D+ACIN)+P>>17+C+CARRYIN
      | (D+ACIN)+P>>17+CARRYIN
      | (D+ACIN)+P>>17+P
      | (D+ACIN)+P>>17+P+CARRYIN
      | (D+ACIN)+PCIN
      | (D+ACIN)+PCIN+C
      | (D+ACIN)+PCIN+C+CARRYIN
      | (D+ACIN)+PCIN+CARRYIN
      | (D+ACIN)+PCIN+P
      | (D+ACIN)+PCIN+P+CARRYIN
      | (D+ACIN)+PCIN>>17
      | (D+ACIN)+PCIN>>17+C
      | (D+ACIN)+PCIN>>17+C+CARRYIN
      | (D+ACIN)+PCIN>>17+CARRYIN
      | (D+ACIN)+PCIN>>17+P
      | (D+ACIN)+PCIN>>17+P+CARRYIN
      | (D+ACIN)-C
      | (D+ACIN)-P
      | (D+ACIN)-P>>17
      | (D+ACIN)-PCIN
      | (D+ACIN)-PCIN>>17
      | (D-A)
      | (D-A)*(D-A)
      | (D-A)*(D-A)+C
      | (D-A)*(D-A)+C+C
      | (D-A)*(D-A)+C+C+CARRYIN
      | (D-A)*(D-A)+C+CARRYIN
      | (D-A)*(D-A)+C+P
      | (D-A)*(D-A)+C+P+CARRYIN
      | (D-A)*(D-A)+C+P>>17
      | (D-A)*(D-A)+C+P>>17+CARRYIN
      | (D-A)*(D-A)+C+PCIN
      | (D-A)*(D-A)+C+PCIN+CARRYIN
      | (D-A)*(D-A)+C+PCIN>>17
      | (D-A)*(D-A)+C+PCIN>>17+CARRYIN
      | (D-A)*(D-A)+C-C
      | (D-A)*(D-A)+C-P
      | (D-A)*(D-A)+C-P>>17
      | (D-A)*(D-A)+C-PCIN
      | (D-A)*(D-A)+C-PCIN>>17
      | (D-A)*(D-A)+CARRYIN
      | (D-A)*(D-A)+P
      | (D-A)*(D-A)+P+C
      | (D-A)*(D-A)+P+C+CARRYIN
      | (D-A)*(D-A)+P+CARRYIN
      | (D-A)*(D-A)+P+P
      | (D-A)*(D-A)+P+P+CARRYIN
      | (D-A)*(D-A)+P+P>>17
      | (D-A)*(D-A)+P+P>>17+CARRYIN
      | (D-A)*(D-A)+P+PCIN
      | (D-A)*(D-A)+P+PCIN+CARRYIN
      | (D-A)*(D-A)+P+PCIN>>17
      | (D-A)*(D-A)+P+PCIN>>17+CARRYIN
      | (D-A)*(D-A)+P-C
      | (D-A)*(D-A)+P-P
      | (D-A)*(D-A)+P-P>>17
      | (D-A)*(D-A)+P-PCIN
      | (D-A)*(D-A)+P-PCIN>>17
      | (D-A)*(D-A)+P>>17
      | (D-A)*(D-A)+P>>17+C
      | (D-A)*(D-A)+P>>17+C+CARRYIN
      | (D-A)*(D-A)+P>>17+CARRYIN
      | (D-A)*(D-A)+P>>17+P
      | (D-A)*(D-A)+P>>17+P+CARRYIN
      | (D-A)*(D-A)+PCIN
      | (D-A)*(D-A)+PCIN+C
      | (D-A)*(D-A)+PCIN+C+CARRYIN
      | (D-A)*(D-A)+PCIN+CARRYIN
      | (D-A)*(D-A)+PCIN+P
      | (D-A)*(D-A)+PCIN+P+CARRYIN
      | (D-A)*(D-A)+PCIN>>17
      | (D-A)*(D-A)+PCIN>>17+C
      | (D-A)*(D-A)+PCIN>>17+C+CARRYIN
      | (D-A)*(D-A)+PCIN>>17+CARRYIN
      | (D-A)*(D-A)+PCIN>>17+P
      | (D-A)*(D-A)+PCIN>>17+P+CARRYIN
      | (D-A)*(D-A)-C
      | (D-A)*(D-A)-P
      | (D-A)*(D-A)-P>>17
      | (D-A)*(D-A)-PCIN
      | (D-A)*(D-A)-PCIN>>17
      | (D-A)*B
      | (D-A)*B+C
      | (D-A)*B+C+C
      | (D-A)*B+C+C+CARRYIN
      | (D-A)*B+C+CARRYIN
      | (D-A)*B+C+P
      | (D-A)*B+C+P+CARRYIN
      | (D-A)*B+C+P>>17
      | (D-A)*B+C+P>>17+CARRYIN
      | (D-A)*B+C+PCIN
      | (D-A)*B+C+PCIN+CARRYIN
      | (D-A)*B+C+PCIN>>17
      | (D-A)*B+C+PCIN>>17+CARRYIN
      | (D-A)*B+C-C
      | (D-A)*B+C-P
      | (D-A)*B+C-P>>17
      | (D-A)*B+C-PCIN
      | (D-A)*B+C-PCIN>>17
      | (D-A)*B+CARRYIN
      | (D-A)*B+P
      | (D-A)*B+P+C
      | (D-A)*B+P+C+CARRYIN
      | (D-A)*B+P+CARRYIN
      | (D-A)*B+P+P
      | (D-A)*B+P+P+CARRYIN
      | (D-A)*B+P+P>>17
      | (D-A)*B+P+P>>17+CARRYIN
      | (D-A)*B+P+PCIN
      | (D-A)*B+P+PCIN+CARRYIN
      | (D-A)*B+P+PCIN>>17
      | (D-A)*B+P+PCIN>>17+CARRYIN
      | (D-A)*B+P-C
      | (D-A)*B+P-P
      | (D-A)*B+P-P>>17
      | (D-A)*B+P-PCIN
      | (D-A)*B+P-PCIN>>17
      | (D-A)*B+P>>17
      | (D-A)*B+P>>17+C
      | (D-A)*B+P>>17+C+CARRYIN
      | (D-A)*B+P>>17+CARRYIN
      | (D-A)*B+P>>17+P
      | (D-A)*B+P>>17+P+CARRYIN
      | (D-A)*B+PCIN
      | (D-A)*B+PCIN+C
      | (D-A)*B+PCIN+C+CARRYIN
      | (D-A)*B+PCIN+CARRYIN
      | (D-A)*B+PCIN+P
      | (D-A)*B+PCIN+P+CARRYIN
      | (D-A)*B+PCIN>>17
      | (D-A)*B+PCIN>>17+C
      | (D-A)*B+PCIN>>17+C+CARRYIN
      | (D-A)*B+PCIN>>17+CARRYIN
      | (D-A)*B+PCIN>>17+P
      | (D-A)*B+PCIN>>17+P+CARRYIN
      | (D-A)*B-C
      | (D-A)*B-P
      | (D-A)*B-P>>17
      | (D-A)*B-PCIN
      | (D-A)*B-PCIN>>17
      | (D-A)*BCIN
      | (D-A)*BCIN+C
      | (D-A)*BCIN+C+C
      | (D-A)*BCIN+C+C+CARRYIN
      | (D-A)*BCIN+C+CARRYIN
      | (D-A)*BCIN+C+P
      | (D-A)*BCIN+C+P+CARRYIN
      | (D-A)*BCIN+C+P>>17
      | (D-A)*BCIN+C+P>>17+CARRYIN
      | (D-A)*BCIN+C+PCIN
      | (D-A)*BCIN+C+PCIN+CARRYIN
      | (D-A)*BCIN+C+PCIN>>17
      | (D-A)*BCIN+C+PCIN>>17+CARRYIN
      | (D-A)*BCIN+C-C
      | (D-A)*BCIN+C-P
      | (D-A)*BCIN+C-P>>17
      | (D-A)*BCIN+C-PCIN
      | (D-A)*BCIN+C-PCIN>>17
      | (D-A)*BCIN+CARRYIN
      | (D-A)*BCIN+P
      | (D-A)*BCIN+P+C
      | (D-A)*BCIN+P+C+CARRYIN
      | (D-A)*BCIN+P+CARRYIN
      | (D-A)*BCIN+P+P
      | (D-A)*BCIN+P+P+CARRYIN
      | (D-A)*BCIN+P+P>>17
      | (D-A)*BCIN+P+P>>17+CARRYIN
      | (D-A)*BCIN+P+PCIN
      | (D-A)*BCIN+P+PCIN+CARRYIN
      | (D-A)*BCIN+P+PCIN>>17
      | (D-A)*BCIN+P+PCIN>>17+CARRYIN
      | (D-A)*BCIN+P-C
      | (D-A)*BCIN+P-P
      | (D-A)*BCIN+P-P>>17
      | (D-A)*BCIN+P-PCIN
      | (D-A)*BCIN+P-PCIN>>17
      | (D-A)*BCIN+P>>17
      | (D-A)*BCIN+P>>17+C
      | (D-A)*BCIN+P>>17+C+CARRYIN
      | (D-A)*BCIN+P>>17+CARRYIN
      | (D-A)*BCIN+P>>17+P
      | (D-A)*BCIN+P>>17+P+CARRYIN
      | (D-A)*BCIN+PCIN
      | (D-A)*BCIN+PCIN+C
      | (D-A)*BCIN+PCIN+C+CARRYIN
      | (D-A)*BCIN+PCIN+CARRYIN
      | (D-A)*BCIN+PCIN+P
      | (D-A)*BCIN+PCIN+P+CARRYIN
      | (D-A)*BCIN+PCIN>>17
      | (D-A)*BCIN+PCIN>>17+C
      | (D-A)*BCIN+PCIN>>17+C+CARRYIN
      | (D-A)*BCIN+PCIN>>17+CARRYIN
      | (D-A)*BCIN+PCIN>>17+P
      | (D-A)*BCIN+PCIN>>17+P+CARRYIN
      | (D-A)*BCIN-C
      | (D-A)*BCIN-P
      | (D-A)*BCIN-P>>17
      | (D-A)*BCIN-PCIN
      | (D-A)*BCIN-PCIN>>17
      | (D-A)+C
      | (D-A)+C+C
      | (D-A)+C+C+CARRYIN
      | (D-A)+C+CARRYIN
      | (D-A)+C+P
      | (D-A)+C+P+CARRYIN
      | (D-A)+C+P>>17
      | (D-A)+C+P>>17+CARRYIN
      | (D-A)+C+PCIN
      | (D-A)+C+PCIN+CARRYIN
      | (D-A)+C+PCIN>>17
      | (D-A)+C+PCIN>>17+CARRYIN
      | (D-A)+C-C
      | (D-A)+C-P
      | (D-A)+C-P>>17
      | (D-A)+C-PCIN
      | (D-A)+C-PCIN>>17
      | (D-A)+CARRYIN
      | (D-A)+P
      | (D-A)+P+C
      | (D-A)+P+C+CARRYIN
      | (D-A)+P+CARRYIN
      | (D-A)+P+P
      | (D-A)+P+P+CARRYIN
      | (D-A)+P+P>>17
      | (D-A)+P+P>>17+CARRYIN
      | (D-A)+P+PCIN
      | (D-A)+P+PCIN+CARRYIN
      | (D-A)+P+PCIN>>17
      | (D-A)+P+PCIN>>17+CARRYIN
      | (D-A)+P-C
      | (D-A)+P-P
      | (D-A)+P-P>>17
      | (D-A)+P-PCIN
      | (D-A)+P-PCIN>>17
      | (D-A)+P>>17
      | (D-A)+P>>17+C
      | (D-A)+P>>17+C+CARRYIN
      | (D-A)+P>>17+CARRYIN
      | (D-A)+P>>17+P
      | (D-A)+P>>17+P+CARRYIN
      | (D-A)+PCIN
      | (D-A)+PCIN+C
      | (D-A)+PCIN+C+CARRYIN
      | (D-A)+PCIN+CARRYIN
      | (D-A)+PCIN+P
      | (D-A)+PCIN+P+CARRYIN
      | (D-A)+PCIN>>17
      | (D-A)+PCIN>>17+C
      | (D-A)+PCIN>>17+C+CARRYIN
      | (D-A)+PCIN>>17+CARRYIN
      | (D-A)+PCIN>>17+P
      | (D-A)+PCIN>>17+P+CARRYIN
      | (D-A)-C
      | (D-A)-P
      | (D-A)-P>>17
      | (D-A)-PCIN
      | (D-A)-PCIN>>17
      | (D-ACIN)
      | (D-ACIN)*(D-ACIN)
      | (D-ACIN)*(D-ACIN)+C
      | (D-ACIN)*(D-ACIN)+C+C
      | (D-ACIN)*(D-ACIN)+C+C+CARRYIN
      | (D-ACIN)*(D-ACIN)+C+CARRYIN
      | (D-ACIN)*(D-ACIN)+C+P
      | (D-ACIN)*(D-ACIN)+C+P+CARRYIN
      | (D-ACIN)*(D-ACIN)+C+P>>17
      | (D-ACIN)*(D-ACIN)+C+P>>17+CARRYIN
      | (D-ACIN)*(D-ACIN)+C+PCIN
      | (D-ACIN)*(D-ACIN)+C+PCIN+CARRYIN
      | (D-ACIN)*(D-ACIN)+C+PCIN>>17
      | (D-ACIN)*(D-ACIN)+C+PCIN>>17+CARRYIN
      | (D-ACIN)*(D-ACIN)+C-C
      | (D-ACIN)*(D-ACIN)+C-P
      | (D-ACIN)*(D-ACIN)+C-P>>17
      | (D-ACIN)*(D-ACIN)+C-PCIN
      | (D-ACIN)*(D-ACIN)+C-PCIN>>17
      | (D-ACIN)*(D-ACIN)+CARRYIN
      | (D-ACIN)*(D-ACIN)+P
      | (D-ACIN)*(D-ACIN)+P+C
      | (D-ACIN)*(D-ACIN)+P+C+CARRYIN
      | (D-ACIN)*(D-ACIN)+P+CARRYIN
      | (D-ACIN)*(D-ACIN)+P+P
      | (D-ACIN)*(D-ACIN)+P+P+CARRYIN
      | (D-ACIN)*(D-ACIN)+P+P>>17
      | (D-ACIN)*(D-ACIN)+P+P>>17+CARRYIN
      | (D-ACIN)*(D-ACIN)+P+PCIN
      | (D-ACIN)*(D-ACIN)+P+PCIN+CARRYIN
      | (D-ACIN)*(D-ACIN)+P+PCIN>>17
      | (D-ACIN)*(D-ACIN)+P+PCIN>>17+CARRYIN
      | (D-ACIN)*(D-ACIN)+P-C
      | (D-ACIN)*(D-ACIN)+P-P
      | (D-ACIN)*(D-ACIN)+P-P>>17
      | (D-ACIN)*(D-ACIN)+P-PCIN
      | (D-ACIN)*(D-ACIN)+P-PCIN>>17
      | (D-ACIN)*(D-ACIN)+P>>17
      | (D-ACIN)*(D-ACIN)+P>>17+C
      | (D-ACIN)*(D-ACIN)+P>>17+C+CARRYIN
      | (D-ACIN)*(D-ACIN)+P>>17+CARRYIN
      | (D-ACIN)*(D-ACIN)+P>>17+P
      | (D-ACIN)*(D-ACIN)+P>>17+P+CARRYIN
      | (D-ACIN)*(D-ACIN)+PCIN
      | (D-ACIN)*(D-ACIN)+PCIN+C
      | (D-ACIN)*(D-ACIN)+PCIN+C+CARRYIN
      | (D-ACIN)*(D-ACIN)+PCIN+CARRYIN
      | (D-ACIN)*(D-ACIN)+PCIN+P
      | (D-ACIN)*(D-ACIN)+PCIN+P+CARRYIN
      | (D-ACIN)*(D-ACIN)+PCIN>>17
      | (D-ACIN)*(D-ACIN)+PCIN>>17+C